simulink model "non_linear_MBS_final"  (R2021b)
settings: Solver=ode15s  StartTime=0  StopTime=30  FixedStep=.00001  RelTol=1e-3
values from neighbouring files (symbols this model uses):
  A_ratio = 1.41    [non_linear_param_MBS_PF_final.m]    (also in non_linear_param_final.m)
  Annulus_area = Bore_area-Rod_area    (= 2356.19449019)    [non_linear_param_MBS_PF_final.m]    (also in non_linear_param_final.m)
  beta_F = 12000e5    (= 1200000000)    [non_linear_param_MBS_PF_final.m]    (also in non_linear_param_final.m)
  Bore_area = pi/4*Bore_diameter^2    (= 3318.30724035)    [non_linear_param_MBS_PF_final.m]    (also in non_linear_param_final.m)
  cyl_c_end = 9e8    (= 900000000)    [non_linear_param_MBS_PF_final.m]    (also in non_linear_param_final.m)
  cyl_c_visc = 1500    [non_linear_param_MBS_PF_final.m]    (also in non_linear_param_final.m)
  cyl_CH_stat = 1/50    (= 0.02)    [non_linear_param_MBS_PF_final.m]    (also in non_linear_param_final.m)
  cyl_F_coul = 75    [non_linear_param_MBS_PF_final.m]    (also in non_linear_param_final.m)
  cyl_FH_stat = 500    [non_linear_param_MBS_PF_final.m]    (also in non_linear_param_final.m)
  cyl_gamma = 250    [non_linear_param_MBS_PF_final.m]    (also in non_linear_param_final.m)
  cyl_k_end = 6e9    (= 6000000000)    [non_linear_param_MBS_PF_final.m]    (also in non_linear_param_final.m)
  cyl_KLi = 0    [non_linear_param_MBS_PF_final.m]    (also in non_linear_param_final.m)
  d_eff_long = sqrt(2*J_sec_long*k_eff_long)    [non_linear_param_MBS_PF_final.m]    (also in non_linear_param_final.m)
  d_eff_short = sqrt(2*J_sec_short*k_eff_short)    [non_linear_param_MBS_PF_final.m]    (also in non_linear_param_final.m)
  DB = 0    [non_linear_param_MBS_PF_final.m]    (also in non_linear_param_final.m = -0.114)
  DR = 1    [non_linear_param_MBS_PF_final.m]    (also in non_linear_param_final.m)
  dr_dcv = 0.7    [non_linear_param_MBS_PF_final.m]    (also in non_linear_param_final.m)
  Eff_Bulk_On = 1    [non_linear_param_MBS_PF_final.m]    (also in non_linear_param_final.m)
  eta_air = 0.7e-2    (= 0.007)    [non_linear_param_MBS_PF_final.m]    (also in non_linear_param_final.m)
  k_cv = 15    [non_linear_param_MBS_PF_final.m]    (also in non_linear_param_final.m)
  k_DCV_PA = 7.85    [non_linear_param_MBS_PF_final.m]    (also in non_linear_param_final.m)
  k_DCV_PB = 7.55    [non_linear_param_MBS_PF_final.m]    (also in non_linear_param_final.m)
  k_DCV_T = 7.85    [non_linear_param_MBS_PF_final.m]    (also in non_linear_param_final.m)
  k_eff_long = E*I/I_sec_long    (= 3050381.94041)    [non_linear_param_MBS_PF_final.m]    (also in non_linear_param_final.m)
  k_eff_short = E*I/I_sec_short    (= 13451432.5427)    [non_linear_param_MBS_PF_final.m]    (also in non_linear_param_final.m)
  k_ocv = 15    [non_linear_param_MBS_PF_final.m]    (also in non_linear_param_final.m)
  K_oil = 1.5e4*0+12000    (= 12000)    [non_linear_param_MBS_PF_final.m]    (also in non_linear_param_final.m)
  k_tanh = 0.005    [non_linear_param_MBS_PF_final.m]    (also in non_linear_param_final.m)
  kappa_air = 1.4    [non_linear_param_MBS_PF_final.m]    (also in non_linear_param_final.m)
  KR = 1    [non_linear_param_MBS_PF_final.m]    (also in non_linear_param_final.m)
  mu_ocv = 10    [non_linear_param_MBS_PF_final.m]    (also in non_linear_param_final.m = 3)
  OL_pa = -0.114    [non_linear_param_MBS_PF_final.m]    (also in non_linear_param_final.m)
  OL_pb = 0.114    [non_linear_param_MBS_PF_final.m]    (also in non_linear_param_final.m)
  p0_A = 62.79    [non_linear_param_MBS_PF_final.m]    (also in non_linear_param_final.m)
  p0_A1 = 1.08    [non_linear_param_MBS_PF_final.m]    (also in non_linear_param_final.m)
  p0_B = 1.213    [non_linear_param_MBS_PF_final.m]    (also in non_linear_param_final.m)
  p_cv_fo = 1    [non_linear_param_MBS_PF_final.m]    (also in non_linear_param_final.m)
  p_cv_set = 2.8    [non_linear_param_MBS_PF_final.m]    (also in non_linear_param_final.m)
  p_ocv_fo = 350    [non_linear_param_MBS_PF_final.m]    (also in non_linear_param_final.m)
  p_ocv_set = 196    [non_linear_param_MBS_PF_final.m]    (also in non_linear_param_final.m = 150)
  p_pc_set = 7    [non_linear_param_MBS_PF_final.m]    (also in non_linear_param_final.m)
  p_R = 1.1    [non_linear_param_MBS_PF_final.m]    (also in non_linear_param_final.m)
  p_S = 180    [non_linear_param_MBS_PF_final.m]    (also in non_linear_param_final.m)
  patm = 1.013e5    (= 101300)    [non_linear_param_MBS_PF_final.m]    (also in non_linear_param_final.m)
  Stroke = 500    [non_linear_param_MBS_PF_final.m]    (also in non_linear_param_final.m)
  t0 = 3    [non_linear_param_MBS_PF_final.m]    (also in non_linear_param_final.m = 1)
  tau_ocv = 0.0889*0+0.001    (= 0.001)    [non_linear_param_MBS_PF_final.m]    (also in non_linear_param_final.m)
  tau_pc = tau_ocv    (= 0.001)    [non_linear_param_MBS_PF_final.m]    (also in non_linear_param_final.m)
  tStepDown = 4.7*0+7.5    (= 7.5)    [non_linear_param_MBS_PF_final.m]    (also in non_linear_param_final.m = 4.6)
  tStepUp = 10.0    (= 10)    [non_linear_param_MBS_PF_final.m]    (also in non_linear_param_final.m = 6.2)
  tWait = 5.0    (= 5)    [non_linear_param_MBS_PF_final.m]    (also in non_linear_param_final.m = 8)
  UL_at = -0.05    [non_linear_param_MBS_PF_final.m]    (also in non_linear_param_final.m)
  UL_bt = 0.05    [non_linear_param_MBS_PF_final.m]    (also in non_linear_param_final.m)
  Up = 1    [non_linear_param_MBS_PF_final.m]    (also in non_linear_param_final.m)
  uS_sp = -0.4    [non_linear_param_MBS_PF_final.m]    (also in non_linear_param_final.m = -0.55)
  V_CH_A = 1.0e-03    (= 0.001)    [non_linear_param_MBS_PF_final.m]    (also in non_linear_param_final.m)
  V_CH_A1 = 0.1e-03    (= 0.0001)    [non_linear_param_MBS_PF_final.m]    (also in non_linear_param_final.m)
  V_CH_B = 1.25e-03    (= 0.00125)    [non_linear_param_MBS_PF_final.m]    (also in non_linear_param_final.m)
  wn_dcv = 30    [non_linear_param_MBS_PF_final.m]    (also in non_linear_param_final.m)
undefined symbols (not defined in the file or in its folder): sigbuilder_block, smiData, uS0, X, Y, Z
libraries: sm_lib x189, nesl_utility x4, eeGeneralControl x2
(part 6 of 6)

== system non_linear_MBS_final/Test/Beam/Dummy2 ==
  ReferenceFrame2 : sm_lib/Frames and Transforms/Reference Frame
  Rigid Transform1 : sm_lib/Frames and Transforms/Rigid Transform  TranslationMethod=Cartesian  TranslationStandardOffset=0  TranslationLengthUnit=m  TranslationStandardAxis=+Z  TranslationCartesianOffset=[0 0 0]  TranslationROffset=0  TranslationROffsetUnits=m  TranslationZOffset=0  TranslationZOffsetUnits=m  TranslationThetaOffset=0  TranslationThetaOffsetUnits=deg  RotationMethod=ArbitraryAxis  RotationAngleUnits=deg  RotationStandardAxis=+Z  RotationAngle=0  RotationArbitraryAxis=[0 1 0]  FollAlignAxisA=+X  BaseAlignAxisA=+Y  FollAlignAxisB=+Y  BaseAlignAxisB=+Z  RotationSequenceAxes=FollowerAxes  RotationSequence=XYX  RotationSequenceAngles=[0 0 0]  RotationSequenceAnglesUnits=deg  RotationMatrix=[1 0 0; 0 1 0; 0 0 1]
  Rigid Transform5 : sm_lib/Frames and Transforms/Rigid Transform  TranslationMethod=Cartesian  TranslationStandardOffset=0  TranslationLengthUnit=m  TranslationStandardAxis=+Z  TranslationCartesianOffset=[0 0 0]  TranslationROffset=0  TranslationROffsetUnits=m  TranslationZOffset=0  TranslationZOffsetUnits=m  TranslationThetaOffset=0  TranslationThetaOffsetUnits=deg  RotationMethod=ArbitraryAxis  RotationAngleUnits=deg  RotationStandardAxis=+Z  RotationAngle=90  RotationArbitraryAxis=[0 1 0]  FollAlignAxisA=+X  BaseAlignAxisA=+Y  FollAlignAxisB=+Y  BaseAlignAxisB=+Z  RotationSequenceAxes=FollowerAxes  RotationSequence=XYX  RotationSequenceAngles=[0 0 0]  RotationSequenceAnglesUnits=deg  RotationMatrix=[1 0 0; 0 1 0; 0 0 1]
  Solid1 : sm_lib/Body Elements/Cylindrical Solid  GraphicAmbientColor=[0.15 0.15 0.15 1.0]  BasedOnType=Density  CenterOfMass=smiData.Solid(19).CoM  CenterOfMassUnits=mm  GraphicDiffuseColor=[0.2 0.6 0.0]  CylinderLength=0.1  CylinderLengthUnits=m  CylinderRadius=0.1  CylinderRadiusUnits=m  Density=1000  DensityUnits=kg/m^3  DoExposeReferenceFrame=on  GraphicEmissiveColor=[0.0 0.0 0.0 1.0]  ExportEntireGeometry=off  SerializedFrames=<Frames/>  MarkerShape=Sphere  MarkerSize=10  GraphicType=None  InertiaType=PointMass  Mass=0.001  MassUnits=kg  MomentsOfInertia=smiData.Solid(19).MoI  MomentsOfInertiaUnits=kg*mm^2  GraphicOpacity=smiData.Solid(19).opacity  ProductsOfInertia=smiData.Solid(19).PoI  ProductsOfInertiaUnits=kg*mm^2  GraphicShininess=75  GraphicSpecularColor=[0.5 0.5 0.5 1.0]  GraphicVisPropType=SimpleVisualProperties
  B : PMIOPort  Side=Left
  F : PMIOPort  Port=2  Side=Right
  connections:
  B/RConn1 -- Rigid Transform1/LConn1
  ReferenceFrame2/RConn1 -- Rigid Transform1/RConn1 -- Rigid Transform5/LConn1 -- Solid1/RConn1
  Rigid Transform5/RConn1 -- F/RConn1

== system non_linear_MBS_final/Test/Beam/Dummy3 ==
  ReferenceFrame2 : sm_lib/Frames and Transforms/Reference Frame
  Rigid Transform1 : sm_lib/Frames and Transforms/Rigid Transform  TranslationMethod=Cartesian  TranslationStandardOffset=0  TranslationLengthUnit=mm  TranslationStandardAxis=+Z  TranslationCartesianOffset=[80 0 0]  TranslationROffset=0  TranslationROffsetUnits=m  TranslationZOffset=0  TranslationZOffsetUnits=m  TranslationThetaOffset=0  TranslationThetaOffsetUnits=deg  RotationMethod=ArbitraryAxis  RotationAngleUnits=deg  RotationStandardAxis=+Z  RotationAngle=0  RotationArbitraryAxis=[0 1 0]  FollAlignAxisA=+X  BaseAlignAxisA=+Y  FollAlignAxisB=+Y  BaseAlignAxisB=+Z  RotationSequenceAxes=FollowerAxes  RotationSequence=XYX  RotationSequenceAngles=[0 0 0]  RotationSequenceAnglesUnits=deg  RotationMatrix=[1 0 0; 0 1 0; 0 0 1]
  Rigid Transform6 : sm_lib/Frames and Transforms/Rigid Transform  TranslationMethod=Cartesian  TranslationStandardOffset=0  TranslationLengthUnit=mm  TranslationStandardAxis=+Z  TranslationCartesianOffset=[0 0 0]  TranslationROffset=0  TranslationROffsetUnits=m  TranslationZOffset=0  TranslationZOffsetUnits=m  TranslationThetaOffset=0  TranslationThetaOffsetUnits=deg  RotationMethod=ArbitraryAxis  RotationAngleUnits=deg  RotationStandardAxis=+Z  RotationAngle=0  RotationArbitraryAxis=[0 1 0]  FollAlignAxisA=+X  BaseAlignAxisA=+Y  FollAlignAxisB=+Y  BaseAlignAxisB=+Z  RotationSequenceAxes=FollowerAxes  RotationSequence=XYX  RotationSequenceAngles=[0 0 0]  RotationSequenceAnglesUnits=deg  RotationMatrix=[1 0 0; 0 1 0; 0 0 1]
  Solid1 : sm_lib/Body Elements/Cylindrical Solid  GraphicAmbientColor=[0.15 0.15 0.15 1.0]  BasedOnType=Density  CenterOfMass=smiData.Solid(19).CoM  CenterOfMassUnits=mm  GraphicDiffuseColor=[0.2 0.6 0.0]  CylinderLength=0.1  CylinderLengthUnits=m  CylinderRadius=0.1  CylinderRadiusUnits=m  Density=1000  DensityUnits=kg/m^3  DoExposeReferenceFrame=on  GraphicEmissiveColor=[0.0 0.0 0.0 1.0]  ExportEntireGeometry=off  SerializedFrames=<Frames/>  MarkerShape=Sphere  MarkerSize=10  GraphicType=None  InertiaType=CalculateFromGeometry  Mass=smiData.Solid(19).mass  MassUnits=kg  MomentsOfInertia=smiData.Solid(19).MoI  MomentsOfInertiaUnits=kg*mm^2  GraphicOpacity=smiData.Solid(19).opacity  ProductsOfInertia=smiData.Solid(19).PoI  ProductsOfInertiaUnits=kg*mm^2  GraphicShininess=75  GraphicSpecularColor=[0.5 0.5 0.5 1.0]  GraphicVisPropType=SimpleVisualProperties
  B : PMIOPort  Side=Left
  F : PMIOPort  Port=2  Side=Right
  connections:
  Rigid Transform1/LConn1 -- B/RConn1
  ReferenceFrame2/RConn1 -- Solid1/RConn1 -- Rigid Transform1/RConn1 -- Rigid Transform6/LConn1
  Rigid Transform6/RConn1 -- F/RConn1

== system non_linear_MBS_final/Test/CB_1/CB_850_1_RIGID ==
  ReferenceFrame : sm_lib/Frames and Transforms/Reference Frame
  Solid : sm_lib/Body Elements/File Solid  ExtGeomFileName=CB_850_Default_sldprt.STEP  UnitType=FromFile  ExtGeomFileUnits=m  StepReaderType=OCC  ExportConvexHullGeometry=off  InertiaType=CalculateFromGeometry  Mass=smiData.Solid(21).mass  MassUnits=kg  CenterOfMass=smiData.Solid(21).CoM  CenterOfMassUnits=mm  MomentsOfInertia=smiData.Solid(21).MoI  MomentsOfInertiaUnits=kg*mm^2  ProductsOfInertia=smiData.Solid(21).PoI  ProductsOfInertiaUnits=kg*mm^2  BasedOnType=Density  Density=8702  DensityUnits=kg/m^3  DoExposeReferenceFrame=on  SerializedFrames=<Frames/>  GraphicType=FromGeometry  MarkerShape=Sphere  MarkerSize=10  GraphicVisPropType=SimpleVisualProperties  GraphicDiffuseColor=[0.0 0.6 1.0]  GraphicSpecularColor=[0.5 0.5 0.5 1.0]  GraphicAmbientColor=[0.15 0.15 0.15 1.0]  GraphicEmissiveColor=[0.0 0.0 0.0 1.0]  GraphicShininess=75  GraphicOpacity=smiData.Solid(21).opacity
  F : PMIOPort  Side=Left
  connections:
  Solid/RConn1 -- ReferenceFrame/RConn1 -- F/RConn1

== system non_linear_MBS_final/Test/CB_1/CB_850_2_RIGID ==
  ReferenceFrame : sm_lib/Frames and Transforms/Reference Frame
  Solid : sm_lib/Body Elements/File Solid  ExtGeomFileName=CB_850_Default_sldprt.STEP  UnitType=FromFile  ExtGeomFileUnits=m  StepReaderType=OCC  ExportConvexHullGeometry=off  InertiaType=CalculateFromGeometry  Mass=smiData.Solid(21).mass  MassUnits=kg  CenterOfMass=smiData.Solid(21).CoM  CenterOfMassUnits=mm  MomentsOfInertia=smiData.Solid(21).MoI  MomentsOfInertiaUnits=kg*mm^2  ProductsOfInertia=smiData.Solid(21).PoI  ProductsOfInertiaUnits=kg*mm^2  BasedOnType=Density  Density=8702  DensityUnits=kg/m^3  DoExposeReferenceFrame=on  SerializedFrames=<Frames/>  GraphicType=FromGeometry  MarkerShape=Sphere  MarkerSize=10  GraphicVisPropType=SimpleVisualProperties  GraphicDiffuseColor=[0.0 0.6 1.0]  GraphicSpecularColor=[0.5 0.5 0.5 1.0]  GraphicAmbientColor=[0.15 0.15 0.15 1.0]  GraphicEmissiveColor=[0.0 0.0 0.0 1.0]  GraphicShininess=75  GraphicOpacity=smiData.Solid(21).opacity
  F : PMIOPort  Side=Left
  connections:
  Solid/RConn1 -- ReferenceFrame/RConn1 -- F/RConn1

== system non_linear_MBS_final/Test/CB_1/CB_plate_1_RIGID ==
  ReferenceFrame : sm_lib/Frames and Transforms/Reference Frame
  Solid : sm_lib/Body Elements/File Solid  ExtGeomFileName=CB_plate_Default_sldprt.STEP  UnitType=FromFile  ExtGeomFileUnits=m  StepReaderType=OCC  ExportConvexHullGeometry=off  InertiaType=CalculateFromGeometry  Mass=smiData.Solid(22).mass  MassUnits=kg  CenterOfMass=smiData.Solid(22).CoM  CenterOfMassUnits=mm  MomentsOfInertia=smiData.Solid(22).MoI  MomentsOfInertiaUnits=kg*mm^2  ProductsOfInertia=smiData.Solid(22).PoI  ProductsOfInertiaUnits=kg*mm^2  BasedOnType=Density  Density=8702  DensityUnits=kg/m^3  DoExposeReferenceFrame=on  SerializedFrames=<Frames/>  GraphicType=FromGeometry  MarkerShape=Sphere  MarkerSize=10  GraphicVisPropType=SimpleVisualProperties  GraphicDiffuseColor=[0.0 0.6 1.0]  GraphicSpecularColor=[0.5 0.5 0.5 1.0]  GraphicAmbientColor=[0.15 0.15 0.15 1.0]  GraphicEmissiveColor=[0.0 0.0 0.0 1.0]  GraphicShininess=75  GraphicOpacity=smiData.Solid(22).opacity
  F : PMIOPort  Side=Left
  connections:
  Solid/RConn1 -- ReferenceFrame/RConn1 -- F/RConn1

== system non_linear_MBS_final/Test/CB_2/CB_850_1_RIGID ==
  ReferenceFrame : sm_lib/Frames and Transforms/Reference Frame
  Solid : sm_lib/Body Elements/File Solid  ExtGeomFileName=CB_850_Default_sldprt.STEP  UnitType=FromFile  ExtGeomFileUnits=m  StepReaderType=OCC  ExportConvexHullGeometry=off  InertiaType=CalculateFromGeometry  Mass=0.001/3  MassUnits=kg  CenterOfMass=smiData.Solid(21).CoM  CenterOfMassUnits=mm  MomentsOfInertia=smiData.Solid(21).MoI  MomentsOfInertiaUnits=kg*mm^2  ProductsOfInertia=smiData.Solid(21).PoI  ProductsOfInertiaUnits=kg*mm^2  BasedOnType=Mass  Density=1000  DensityUnits=kg/m^3  DoExposeReferenceFrame=on  SerializedFrames=<Frames/>  GraphicType=FromGeometry  MarkerShape=Sphere  MarkerSize=10  GraphicVisPropType=SimpleVisualProperties  GraphicDiffuseColor=[0.0 0.6 1.0]  GraphicSpecularColor=[0.5 0.5 0.5 1.0]  GraphicAmbientColor=[0.15 0.15 0.15 1.0]  GraphicEmissiveColor=[0.0 0.0 0.0 1.0]  GraphicShininess=75  GraphicOpacity=smiData.Solid(21).opacity
  F : PMIOPort  Side=Left
  connections:
  Solid/RConn1 -- ReferenceFrame/RConn1 -- F/RConn1

== system non_linear_MBS_final/Test/CB_2/CB_850_2_RIGID ==
  ReferenceFrame : sm_lib/Frames and Transforms/Reference Frame
  Solid : sm_lib/Body Elements/File Solid  ExtGeomFileName=CB_850_Default_sldprt.STEP  UnitType=FromFile  ExtGeomFileUnits=m  StepReaderType=OCC  ExportConvexHullGeometry=off  InertiaType=CalculateFromGeometry  Mass=0.001/3  MassUnits=kg  CenterOfMass=smiData.Solid(21).CoM  CenterOfMassUnits=mm  MomentsOfInertia=smiData.Solid(21).MoI  MomentsOfInertiaUnits=kg*mm^2  ProductsOfInertia=smiData.Solid(21).PoI  ProductsOfInertiaUnits=kg*mm^2  BasedOnType=Mass  Density=1000  DensityUnits=kg/m^3  DoExposeReferenceFrame=on  SerializedFrames=<Frames/>  GraphicType=FromGeometry  MarkerShape=Sphere  MarkerSize=10  GraphicVisPropType=SimpleVisualProperties  GraphicDiffuseColor=[0.0 0.6 1.0]  GraphicSpecularColor=[0.5 0.5 0.5 1.0]  GraphicAmbientColor=[0.15 0.15 0.15 1.0]  GraphicEmissiveColor=[0.0 0.0 0.0 1.0]  GraphicShininess=75  GraphicOpacity=smiData.Solid(21).opacity
  F : PMIOPort  Side=Left
  connections:
  Solid/RConn1 -- ReferenceFrame/RConn1 -- F/RConn1

== system non_linear_MBS_final/Test/CB_2/CB_plate_1_RIGID ==
  ReferenceFrame : sm_lib/Frames and Transforms/Reference Frame
  Solid : sm_lib/Body Elements/File Solid  ExtGeomFileName=CB_plate_Default_sldprt.STEP  UnitType=FromFile  ExtGeomFileUnits=m  StepReaderType=OCC  ExportConvexHullGeometry=off  InertiaType=CalculateFromGeometry  Mass=0.001/3  MassUnits=kg  CenterOfMass=smiData.Solid(22).CoM  CenterOfMassUnits=mm  MomentsOfInertia=smiData.Solid(22).MoI  MomentsOfInertiaUnits=kg*mm^2  ProductsOfInertia=smiData.Solid(22).PoI  ProductsOfInertiaUnits=kg*mm^2  BasedOnType=Mass  Density=1000  DensityUnits=kg/m^3  DoExposeReferenceFrame=on  SerializedFrames=<Frames/>  GraphicType=FromGeometry  MarkerShape=Sphere  MarkerSize=10  GraphicVisPropType=SimpleVisualProperties  GraphicDiffuseColor=[0.0 0.6 1.0]  GraphicSpecularColor=[0.5 0.5 0.5 1.0]  GraphicAmbientColor=[0.15 0.15 0.15 1.0]  GraphicEmissiveColor=[0.0 0.0 0.0 1.0]  GraphicShininess=75  GraphicOpacity=smiData.Solid(22).opacity
  F : PMIOPort  Side=Left
  connections:
  Solid/RConn1 -- ReferenceFrame/RConn1 -- F/RConn1

== system non_linear_MBS_final/Directional Control Valve/Edge Flows/Flow Characteristics/Sign ==
  In : Inport
  Gain2 : Gain  Gain=1/k_tanh
  Trigonometric Function : Trigonometry  Operator=tanh
  Out : Outport  VectorParamsAs1DForOutWhenUnconnected=off
  wires:
  In/1 -> Gain2/1
  Gain2/1 -> Trigonometric Function/1
  Trigonometric Function/1 -> Out/1  "[-]"

== system non_linear_MBS_final/Directional Control Valve/Edge Flows/Flow Characteristics1/Sign ==
  In : Inport
  Gain2 : Gain  Gain=1/k_tanh
  Trigonometric Function : Trigonometry  Operator=tanh
  Out : Outport  VectorParamsAs1DForOutWhenUnconnected=off
  wires:
  In/1 -> Gain2/1
  Gain2/1 -> Trigonometric Function/1
  Trigonometric Function/1 -> Out/1  "[-]"

== system non_linear_MBS_final/Directional Control Valve/Edge Flows/Flow Characteristics2/Sign ==
  In : Inport
  Gain2 : Gain  Gain=1/k_tanh
  Trigonometric Function : Trigonometry  Operator=tanh
  Out : Outport  VectorParamsAs1DForOutWhenUnconnected=off
  wires:
  In/1 -> Gain2/1
  Gain2/1 -> Trigonometric Function/1
  Trigonometric Function/1 -> Out/1  "[-]"

== system non_linear_MBS_final/Directional Control Valve/Edge Flows/Flow Characteristics3/Sign ==
  In : Inport
  Gain2 : Gain  Gain=1/k_tanh
  Trigonometric Function : Trigonometry  Operator=tanh
  Out : Outport  VectorParamsAs1DForOutWhenUnconnected=off
  wires:
  In/1 -> Gain2/1
  Gain2/1 -> Trigonometric Function/1
  Trigonometric Function/1 -> Out/1  "[-]"

== system non_linear_MBS_final/Over-Centre Valve/Capasitance/Bulk Modulus/Effective Bulk Modulus ==
  2 : Scope
  MATLAB Function : MATLAB Function  TreatAsAtomicUnit=on
  K_oil_eff : Outport  VectorParamsAs1DForOutWhenUnconnected=off
  wires:
  MATLAB Function/1(K_oil_eff) -> K_oil_eff/1, 2/1
  [p_A1] -> MATLAB Function/1(p)

== system non_linear_MBS_final/Subsystem/CH Bore Side/Bulk Modulus/Effective Bulk Modulus ==
  2 : Scope
  MATLAB Function : MATLAB Function  TreatAsAtomicUnit=on
  K_oil_eff : Outport  VectorParamsAs1DForOutWhenUnconnected=off
  wires:
  MATLAB Function/1(K_oil_eff) -> K_oil_eff/1, 2/1
  [p_A] -> MATLAB Function/1(p)

== system non_linear_MBS_final/Subsystem/CH Rod Side/Bulk Modulus/Effective Bulk Modulus ==
  2 : Scope
  MATLAB Function : MATLAB Function  TreatAsAtomicUnit=on
  K_oil_eff : Outport  VectorParamsAs1DForOutWhenUnconnected=off
  wires:
  MATLAB Function/1(K_oil_eff) -> K_oil_eff/1, 2/1
  [p_B] -> MATLAB Function/1(p)

== system non_linear_MBS_final/Test/Base/Base_bearing_mount_1_RIGID/Base_bearing_mount_sideways_1_RIGID ==
  ReferenceFrame : sm_lib/Frames and Transforms/Reference Frame
  Solid : sm_lib/Body Elements/File Solid  ExtGeomFileName=Base_bearing_mount_sideways_Default_sldprt.STEP  UnitType=FromFile  ExtGeomFileUnits=m  StepReaderType=HEX  ExportConvexHullGeometry=off  InertiaType=Custom  Mass=smiData.Solid(6).mass  MassUnits=kg  CenterOfMass=smiData.Solid(6).CoM  CenterOfMassUnits=mm  MomentsOfInertia=smiData.Solid(6).MoI  MomentsOfInertiaUnits=kg*mm^2  ProductsOfInertia=smiData.Solid(6).PoI  ProductsOfInertiaUnits=kg*mm^2  BasedOnType=Density  Density=1000  DensityUnits=kg/m^3  DoExposeReferenceFrame=on  SerializedFrames=<Frames/>  GraphicType=FromGeometry  MarkerShape=Sphere  MarkerSize=10  GraphicVisPropType=SimpleVisualProperties  GraphicDiffuseColor=smiData.Solid(6).color  GraphicSpecularColor=[0.5 0.5 0.5 1.0]  GraphicAmbientColor=[0.15 0.15 0.15 1.0]  GraphicEmissiveColor=[0.0 0.0 0.0 1.0]  GraphicShininess=75  GraphicOpacity=smiData.Solid(6).opacity
  F : PMIOPort  Side=Left
  connections:
  Solid/RConn1 -- ReferenceFrame/RConn1 -- F/RConn1

== system non_linear_MBS_final/Test/Base/Base_bearing_mount_1_RIGID/Base_bearing_mount_support_1_RIGID ==
  ReferenceFrame : sm_lib/Frames and Transforms/Reference Frame
  Solid : sm_lib/Body Elements/File Solid  ExtGeomFileName=Base_bearing_mount_support_Default_sldprt.STEP  UnitType=FromFile  ExtGeomFileUnits=m  StepReaderType=HEX  ExportConvexHullGeometry=off  InertiaType=Custom  Mass=smiData.Solid(7).mass  MassUnits=kg  CenterOfMass=smiData.Solid(7).CoM  CenterOfMassUnits=mm  MomentsOfInertia=smiData.Solid(7).MoI  MomentsOfInertiaUnits=kg*mm^2  ProductsOfInertia=smiData.Solid(7).PoI  ProductsOfInertiaUnits=kg*mm^2  BasedOnType=Density  Density=1000  DensityUnits=kg/m^3  DoExposeReferenceFrame=on  SerializedFrames=<Frames/>  GraphicType=FromGeometry  MarkerShape=Sphere  MarkerSize=10  GraphicVisPropType=SimpleVisualProperties  GraphicDiffuseColor=smiData.Solid(7).color  GraphicSpecularColor=[0.5 0.5 0.5 1.0]  GraphicAmbientColor=[0.15 0.15 0.15 1.0]  GraphicEmissiveColor=[0.0 0.0 0.0 1.0]  GraphicShininess=75  GraphicOpacity=smiData.Solid(7).opacity
  F : PMIOPort  Side=Left
  connections:
  Solid/RConn1 -- ReferenceFrame/RConn1 -- F/RConn1

== system non_linear_MBS_final/Test/Base/Base_bearing_mount_1_RIGID/Base_bearing_mount_support_2_RIGID ==
  ReferenceFrame : sm_lib/Frames and Transforms/Reference Frame
  Solid : sm_lib/Body Elements/File Solid  ExtGeomFileName=Base_bearing_mount_support_Default_sldprt.STEP  UnitType=FromFile  ExtGeomFileUnits=m  StepReaderType=HEX  ExportConvexHullGeometry=off  InertiaType=Custom  Mass=smiData.Solid(7).mass  MassUnits=kg  CenterOfMass=smiData.Solid(7).CoM  CenterOfMassUnits=mm  MomentsOfInertia=smiData.Solid(7).MoI  MomentsOfInertiaUnits=kg*mm^2  ProductsOfInertia=smiData.Solid(7).PoI  ProductsOfInertiaUnits=kg*mm^2  BasedOnType=Density  Density=1000  DensityUnits=kg/m^3  DoExposeReferenceFrame=on  SerializedFrames=<Frames/>  GraphicType=FromGeometry  MarkerShape=Sphere  MarkerSize=10  GraphicVisPropType=SimpleVisualProperties  GraphicDiffuseColor=smiData.Solid(7).color  GraphicSpecularColor=[0.5 0.5 0.5 1.0]  GraphicAmbientColor=[0.15 0.15 0.15 1.0]  GraphicEmissiveColor=[0.0 0.0 0.0 1.0]  GraphicShininess=75  GraphicOpacity=smiData.Solid(7).opacity
  F : PMIOPort  Side=Left
  connections:
  Solid/RConn1 -- ReferenceFrame/RConn1 -- F/RConn1

== matlab function non_linear_MBS_final/Directional Control Valve/Edge Openings/Edge P-A/MATLAB Function1 ==
  data: u_dcv input; u_PA output; OL_pa parameter; OL_pb parameter; UL_at parameter; UL_bt parameter
function u_PA = fcn(u_dcv,OL_pa, OL_pb, UL_at, UL_bt)

  u_PA = 0;

if OL_pa <=  u_dcv 
 u_PA = 0;
 
elseif u_dcv <  OL_pa
  u_PA= -(u_dcv-OL_pa)/(1+OL_pa);
end

== matlab function non_linear_MBS_final/Directional Control Valve/Edge Openings/Edge P-B/MATLAB Function1 ==
  data: u_dcv input; u_PB output; OL_pa parameter; OL_pb parameter; UL_at parameter; UL_bt parameter
function u_PB = fcn(u_dcv,OL_pa, OL_pb, UL_at, UL_bt)

  u_PB = 0;

if u_dcv <=   OL_pb
 u_PB = 0;
 
elseif  OL_pb < u_dcv 
  u_PB = (u_dcv-OL_pb)/(1-OL_pb);
end

== matlab function non_linear_MBS_final/Directional Control Valve/Load-Sensing ==
  data: p_R input; p_B input; p_A1 input; u_dcv input; p_LS output
function p_LS  = fcn(p_R,p_B,p_A1,u_dcv)

p_LS=p_R;

if u_dcv==0
%     if p_A1 > p_B
%     p_LS=p_A1;
%     else
%         p_LS=p_B;
%     end

p_LS = p_R;

end

if u_dcv > 0
    p_LS=p_B;
end

if u_dcv < 0
    p_LS=p_A1;
end
end

== matlab function non_linear_MBS_final/Over-Centre Valve/Capasitance/Bulk Modulus/Effective Bulk Modulus/MATLAB Function ==
  data: p input; K_oil_eff output; patm parameter; eta_air parameter; kappa_air parameter; beta_F parameter
function K_oil_eff = fcn(p,patm, eta_air, kappa_air, beta_F)
p = p*1e5;
% Effective bulk modulus 
beta_eff_Pa  = 1/(1/beta_F+1/(((1-eta_air)*(patm/(p+patm))^(-1/kappa_air)/eta_air+1)*kappa_air*(p+patm)));
K_oil_eff = beta_eff_Pa*1e-5;

== matlab function non_linear_MBS_final/Subsystem/CH Bore Side/Bulk Modulus/Effective Bulk Modulus/MATLAB Function ==
  data: p input; K_oil_eff output; patm parameter; eta_air parameter; kappa_air parameter; beta_F parameter
function K_oil_eff = fcn(p,patm, eta_air, kappa_air, beta_F)
p = p*1e5;
% Effective bulk modulus 
beta_eff_Pa  = 1/(1/beta_F+1/(((1-eta_air)*(patm/(p+patm))^(-1/kappa_air)/eta_air+1)*kappa_air*(p+patm)));
K_oil_eff = beta_eff_Pa*1e-5;

== matlab function non_linear_MBS_final/Subsystem/CH Rod Side/Bulk Modulus/Effective Bulk Modulus/MATLAB Function ==
  data: p input; K_oil_eff output; patm parameter; eta_air parameter; kappa_air parameter; beta_F parameter
function K_oil_eff = fcn(p,patm, eta_air, kappa_air, beta_F)
p = p*1e5;
% Effective bulk modulus 
beta_eff_Pa  = 1/(1/beta_F+1/(((1-eta_air)*(patm/(p+patm))^(-1/kappa_air)/eta_air+1)*kappa_air*(p+patm)));
K_oil_eff = beta_eff_Pa*1e-5;

== matlab function non_linear_MBS_final/Directional Control Valve/Edge Openings/Edge A-T/MATLAB Function1 ==
  data: u_dcv input; u_AT output; OL_pa parameter; OL_pb parameter; UL_at parameter; UL_bt parameter
function u_AT = fcn(u_dcv,OL_pa, OL_pb, UL_at, UL_bt)

u_AT = 0;

if u_dcv <= UL_at
 u_AT = 0;
 
elseif u_dcv > UL_at   && u_dcv < 2*OL_pb
 u_AT= ((u_dcv-UL_at)*OL_pb)/((1-OL_pb)*(-UL_at+2*OL_pb));
 
elseif 2*OL_pb <= u_dcv
  u_AT= (u_dcv-OL_pb)/(1-OL_pb);
end

== matlab function non_linear_MBS_final/Step Input Generator/VCC_Step_Function_Validation/StepFunction ==
  data: u_SP input; uS0 input; DB input; t input; t0 input; t1 input; t2 input; y output; T output; t3 input
function [y, T] = step(u_SP, uS0, DB,  t, t0,t1,t2,t3)
y = 0;



if t>=0 && t<t0
    y = uS0;
elseif t>=(t0) && t<(t0+t1)
    y = u_SP+DB; 
elseif t>=(t0+t1) && t<(t0+t1+t2)
    y = uS0; 
elseif t>=(t0+t1+t2) && t<(t0+t1+t2+t3)
    y = -u_SP-DB;          
else
    y = 0; 
end


T = t0+t1+t2+t3;

== matlab function non_linear_MBS_final/Directional Control Valve/Edge Openings/Edge B-T/MATLAB Function ==
  data: u_dcv input; u_BT output; OL_pa parameter; OL_pb parameter; UL_at parameter; UL_bt parameter
function u_BT = fcn(u_dcv,OL_pa, OL_pb, UL_at, UL_bt)

  u_BT = 0;

if UL_bt <=  u_dcv 
 u_BT = 0;
 
elseif 2*OL_pa < u_dcv && u_dcv < UL_bt
 u_BT= ((u_dcv-UL_bt)*OL_pa)/((1+OL_pa)*(UL_bt-2*OL_pa));
 
elseif u_dcv <=  2*OL_pa
  u_BT= -(u_dcv-OL_pa)/(1+OL_pa);
end

== matlab function non_linear_MBS_final/Step Input Generator/VCC_Step_Function_Validation1/StepFunction ==
  data: u_SP input; uS0 input; DB input; t input; t0 input; t1 input; t2 input; y output; T output; t3 input
function [y, T] = step(u_SP, uS0, DB,  t, t0,t1,t2,t3)
y = 0;



if t>=0 && t<t0
    y = uS0;
elseif t>=(t0) && t<(t0+t1)
    y = u_SP+DB; 
elseif t>=(t0+t1) && t<(t0+t1+t2)
    y = uS0; 
elseif t>=(t0+t1+t2) && t<(t0+t1+t2+t3)
    y = -u_SP-DB;          
else
    y = 0; 
end


T = t0+t1+t2+t3;

== matlab function non_linear_MBS_final/Subsystem1/VCC_Step_Function_Validation/StepFunction ==
  data: u_SP input; uS0 input; DB input; t input; t0 input; t1 input; t2 input; y output; T output; t3 input
function [y, T] = step(u_SP, uS0, DB,  t, t0,t1,t2,t3)
y = 0;



if t>=0 && t<t0
    y = uS0;
elseif t>=(t0) && t<(t0+t1)
    y = u_SP+DB; 
elseif t>=(t0+t1) && t<(t0+t1+t2)
    y = uS0; 
elseif t>=(t0+t1+t2) && t<(t0+t1+t2+t3)
    y = -u_SP-DB;          
else
    y = 0; 
end


T = t0+t1+t2+t3;

== matlab function non_linear_MBS_final/Pressure Compensator1/Compensator From Morten Bak Paper ==
  data: p0 input; p_C output; p_S input; p_LS input
function p_C = fcn(p0, p_S, p_LS)

p_C = p_LS;

if p0 <= (p_S-p_LS)
p_C = p_LS+p0;
elseif 0<(p_S-p_LS) < p0
p_C = p_S - p_LS; 
elseif (p_S-p_LS) <= 0
    p_C = p_LS;
end
